simulink model "BMSModel"  (R2024b) (continued)
== system BMSModel/Electric Vehicul Model/Contactors == (continued)
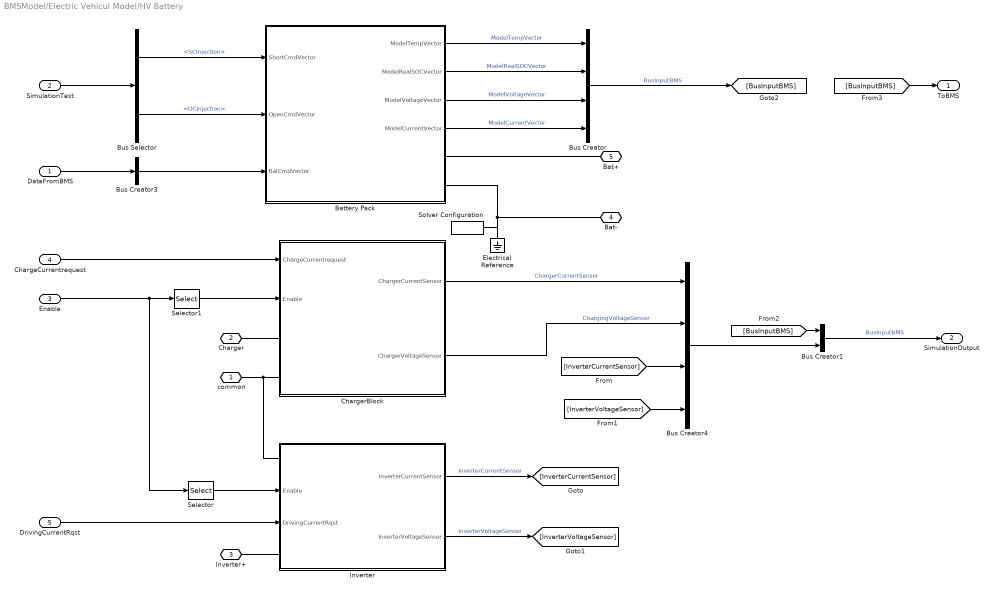
== system BMSModel/Electric Vehicul Model/HV Battery ==
  DataFromBMS : Inport
  SimulationTest : Inport  Port=2
  Enable : Inport  Port=3
  ChargeCurrentrequest : Inport  Port=4
  DrivingCurrentRqst : Inport  Port=5
  Battery Pack : Subsystem
  Bus Creator : BusCreator  Inputs=4
  Bus Creator1 : BusCreator  Inputs=2
  Bus Creator3 : BusCreator  Inputs=1
  Bus Creator4 : BusCreator  Inputs=4
  Bus Selector : BusSelector  OutputSignals=SimulationBatteryPackInput.SCInjection,SimulationBatteryPackInput.OCInjection
  ChargerBlock : Subsystem
  Electrical Reference : fl_lib/Electrical/Electrical Elements/Electrical Reference
  Inverter : Subsystem
      settings: PortSchema="{\"entries\":[{\"content\":{\"sides\":[{\"content\":{\"connectorIds\":[\"LConn1\",\"In1\",\"In2\",\"LConn2\"]},\"type\":\"ConnectorPlacement.EquallySpacedRectSide\",\"uuid\":\"3d4faee9-a6b8-4949-a26b-5ad71604b134\"},{\"content\":{\"connectorIds\":[\"Out1\",\"Out2\"],\"side\":\"RIGHT\"},\"type\":\"ConnectorPlacement.EquallySpacedRectSide\",\"uuid\":\"3f236c3c-2e31-4720-8762-4bdcf15f44c6\"},{\"content\":{\"side\":\"TOP\"},\"type\":\"ConnectorPlacement.EquallySpacedRectSide\",\"uuid\":\"f5349ad7-caad-4613-84e6-ed68add5efa8\"}]},\"type\":\"ConnectorPlacement.EquallySpacedConnector\",\"uuid\":\"6d42bc58-15ab-4454-81b3-fca0047b1a2e\"}],\"packageUris\":[\"http://schema.mathworks.com/mf0/Connector/R2020b\"],\"version\":\"1.0\"}"
  Selector : Selector  InputPortWidth=2  IndexOptions=Index vector (dialog)  Indices=2  OutputSizes=1
  Selector1 : Selector  InputPortWidth=2  IndexOptions=Index vector (dialog)  Indices=1  OutputSizes=1
  Solver Configuration : nesl_utility/Solver Configuration  PhysicalDomain=network_engine_domain  SubClassName=solver  LeftPortType=input  RightPortType=generic  Tones=[]  Harmonics=[]  SimulateNoise=off  Profile=off  UseLocalSampling=off  EnableSwitchedLinearOptims=on  FrequencyDomain=off  UseCCode=off  RelTol=0.001  AbsTol=1e-06  MinStep=1e-09  ToWorkspace=off  RevertToSquareIcSolve=off  NumHomotopyIterations=100  PhasorMode=off  PhasorModeNumHarmonics=1  ConsistencySolver=NEWTON_FTOL  IndexReductionMethod=DerivativeReplacement  DoDC=off  ResidualTolerance=1e-09  ConsistencyTolSource=GLOBAL  ConsistencyAbsTol=0.001  ConsistencyRelTol=0.001  ConsistencyTolFactor=0.001  UseLocalSolver=off  LocalSolverChoice=NE_BACKWARD_EULER_ADVANCER  ResolveIndetEquations=on  FunctionEvalNumThread=1  LocalSolverSampleTime=0.001  DoFixedCost=off  MaxNonlinIter=3  MaxModeIter=2  ComputeImpulses=off  ImpulseIterations=2  LinearAlgebra=auto  LinearAlgebraNumThread=0  EquationFormulation=NE_TIME_EF  DelaysMemoryBudget=1024  AutomaticFiltering=on  FilteringTimeConstant=0.001  PartitionStorageMethod=AS_NEEDED  PartitionMemoryBudget=1024  PartitionMethod=ROBUST  MultibodyUseLocalSolver=off  MultibodyLocalSolverChoice=ODE1  MultibodyLocalSolverSampleTime=0.001
  common : PMIOPort  Side=Left
  Charger : PMIOPort  Port=2  Side=Left
  Inverter+ : PMIOPort  Port=3  Side=Left
  Bat- : PMIOPort  Port=4  Side=Left
  Bat+ : PMIOPort  Port=5  Side=Left
  ToBMS : Outport
  SimulationOutput : Outport  Port=2
  wires:
  Battery Pack/2(ModelRealSOCVector) -> Bus Creator/2  "ModelRealSOCVector"
  Battery Pack/1(ModelTempVector) -> Bus Creator/1  "ModelTempVector"
  Bus Creator1/1 -> SimulationOutput/1  "BusInputBMS"
  Bus Selector/2 -> Battery Pack/2(OpenCmdVector)  "<OCInjection>"
  Battery Pack/4(ModelCurrentVector) -> Bus Creator/4  "ModelCurrentVector"
  Bus Selector/1 -> Battery Pack/1(ShortCmdVector)  "<SCInjection>"
  Battery Pack/3(ModelVoltageVector) -> Bus Creator/3  "ModelVoltageVector"
  Bus Creator/1 -> [BusInputBMS]  "BusInputBMS"
  SimulationTest/1 -> Bus Selector/1
  DataFromBMS/1 -> Bus Creator3/1
  Bus Creator3/1 -> Battery Pack/3(BalCmdVector)
  ChargerBlock/1(ChargerCurrentSensor) -> Bus Creator4/1  "ChargerCurrentSensor"
  ChargerBlock/2(ChargerVoltageSensor) -> Bus Creator4/2  "ChargingVoltageSensor"
  Inverter/1(InverterCurrentSensor) -> [InverterCurrentSensor]  "InverterCurrentSensor"
  Inverter/2(InverterVoltageSensor) -> [InverterVoltageSensor]  "InverterVoltageSensor"
  ChargeCurrentrequest/1 -> ChargerBlock/1(ChargeCurrentrequest)
  DrivingCurrentRqst/1 -> Inverter/2(DrivingCurrentRqst)
  Selector/1 -> Inverter/1(Enable)
  Selector1/1 -> ChargerBlock/2(Enable)
  Enable/1 -> Selector/1, Selector1/1
  Bus Creator4/1 -> Bus Creator1/2
  [InverterCurrentSensor] -> Bus Creator4/3
  [InverterVoltageSensor] -> Bus Creator4/4
  [BusInputBMS] -> Bus Creator1/1
  [BusInputBMS] -> ToBMS/1
  connections:
  Inverter/LConn1 -- common/RConn1 -- ChargerBlock/LConn2
  Charger/RConn1 -- ChargerBlock/LConn1
  Inverter+/RConn1 -- Inverter/LConn2
  Bat+/RConn1 -- Battery Pack/RConn1
  Bat-/RConn1 -- Solver Configuration/RConn1 -- Electrical Reference/LConn1 -- Battery Pack/RConn2

== system BMSModel/Test Senarois/Signal Builder ==
  Demux : Demux  Tag=STV Demux  Outputs=1
      settings: DisplayOption=none
  FromWs : FromWorkspace  Tag=STV FromWs  VariableName=tuvar
      SigBuilderData=|
      | common.afterFinalStr = 'Extrapolate'
      | common.sampleTime = 0
      | dataSet.timeRange = [0 2474]
      | dataSet.name = 'FTP-75 Drive Cycle'
      | sbobj.Groups.Name = 'FTP-75 Drive Cycle'
      | sbobj.Groups.Signals.Name = 'Current'
      | sbobj.Groups.Signals.XData = [0 0.00166966283167 0.00489865696245 0.00887290141926 0.01 0.0132228027433 0.0164456054866 0.0196684082299 0.02 0.0216579588505 0.0216579588505 0.023878217756 ... (264079 elements, 1x264079)]
      | sbobj.Groups.Signals.YData = [0 0 0 0 0 0 0 0 0 0 -7.47487403974e-31 -1.06202348499e-17 ... (264079 elements, 1x264079)]
      | sbobj.Groups.TimeRange = [0 2474]
  Current : Outport  Tag=STV Outport
  wires:
  FromWs/1 -> Demux/1
  Demux/1 -> Current/1

== system BMSModel/Electric Vehicul Model/BMS model/Balancing system ==
  SystemState : Inport
  VoltageVector : Inport  Port=2
  Balancing Function : MATLAB Function
      settings: MinAlgLoopOccurrences=on
  Data Type Conversion : DataTypeConversion  OutDataTypeStr=boolean  SaturateOnIntegerOverflow=off  RndMeth=Floor
  balancingCmd : Outport
  balancingDeltaVCell : Outport  Port=2
  balancingFlags : Outport  Port=3
  wires:
  SystemState/1 -> Balancing Function/1(systemState)
  VoltageVector/1 -> Balancing Function/2(voltageVector)
  Balancing Function/1(balancingCmd) -> Data Type Conversion/1
  Balancing Function/2(balancingFlags) -> balancingFlags/1
  Balancing Function/3(balancingDeltaVCell) -> balancingDeltaVCell/1
  Data Type Conversion/1 -> balancingCmd/1

== system BMSModel/Electric Vehicul Model/BMS model/Contactor commands ==
  SystemState : Inport
  ContactorModuleData : Inport  Port=2
  Bus Creator1 : BusCreator  Inputs=ContactorCmd,PreChrgCmd,DisChrgCmd,DrivetrainEn
  Bus Selector : BusSelector  OutputSignals=VCharger,VInverter,VBatt
  Chart : Stateflow chart  TreatAsAtomicUnit=on
  Data Type Conversion{,1..3} (4 alike) : DataTypeConversion  OutDataTypeStr=double  SaturateOnIntegerOverflow=off  RndMeth=Floor
  Vector Concatenate : Concatenate
  Vector Concatenate1 : Concatenate  NumInputs=3
  Vector Concatenate2 : Concatenate
  Vector Concatenate3 : Concatenate
  ContactorModuleCmd : Outport
  wires:
  Data Type Conversion2/1 -> Bus Creator1/3  "DisChrgCmd"
  Data Type Conversion/1 -> Bus Creator1/2  "PreChrgCmd"
  Vector Concatenate1/1 -> Data Type Conversion1/1
  Chart/4(preChargerCmd) -> Vector Concatenate/1
  Chart/7(disInverterCmd) -> Vector Concatenate2/2
  Vector Concatenate3/1 -> Data Type Conversion3/1
  Vector Concatenate/1 -> Data Type Conversion/1
  Chart/3(contCommonCmd) -> Vector Concatenate1/3
  Chart/6(disChargerCmd) -> Vector Concatenate2/1
  Vector Concatenate2/1 -> Data Type Conversion2/1
  Chart/9(driveEn) -> Vector Concatenate3/2
  SystemState/1 -> Chart/4(systemState)
  Chart/8(chargeEn) -> Vector Concatenate3/1
  Data Type Conversion3/1 -> Bus Creator1/4  "DrivetrainEn"
  Data Type Conversion1/1 -> Bus Creator1/1  "ContactorCmd"
  Chart/1(contChargerCmd) -> Vector Concatenate1/1
  Chart/5(preInverterCmd) -> Vector Concatenate/2
  Bus Creator1/1 -> ContactorModuleCmd/1
  Chart/2(contInverterCmd) -> Vector Concatenate1/2
  ContactorModuleData/1 -> Bus Selector/1
  Bus Selector/1 -> Chart/1(VCharger)  "<VCharger>"
  Bus Selector/2 -> Chart/2(VInverter)  "<VInverter>"
  Bus Selector/3 -> Chart/3(Vbatt)  "<VBatt>"

== system BMSModel/Electric Vehicul Model/BMS model/Faults detection ==
  <CurrentVector> : Inport
  <TempVector> : Inport  Port=2
  <VoltageVector> : Inport  Port=3
  Bus Creator2 : BusCreator  Inputs=8
  MATLAB Function : MATLAB Function  TreatAsAtomicUnit=on
  MATLAB Function1 : MATLAB Function  TreatAsAtomicUnit=on
  MATLAB Function2 : MATLAB Function  TreatAsAtomicUnit=on
  MATLAB Function3 : MATLAB Function  TreatAsAtomicUnit=on
  Max10 : MinMax  Function=max
  Max13 : MinMax
  Max4 : MinMax  Function=max
  Max7 : MinMax  Function=max
  BMSFaultOutput : Outport
  wires:
  Bus Creator2/1 -> BMSFaultOutput/1
  MATLAB Function2/1(W) -> Bus Creator2/3  "OverTemperatureWarning"
  Max4/1 -> MATLAB Function3/1(maxInput)
  <CurrentVector>/1 -> Max4/1
  MATLAB Function3/1(W) -> Bus Creator2/1  "OverCurrentWarning"
  MATLAB Function3/2(F) -> Bus Creator2/2  "OverCurrentFault"
  Max13/1 -> MATLAB Function/1(minInput)
  MATLAB Function1/2(F) -> Bus Creator2/6  "OverVoltageFault"
  MATLAB Function1/1(W) -> Bus Creator2/5  "OverVoltageWarning"
  <TempVector>/1 -> Max7/1
  Max10/1 -> MATLAB Function1/1(maxInput)
  <VoltageVector>/1 -> Max13/1, Max10/1
  Max7/1 -> MATLAB Function2/1(maxInput)
  MATLAB Function/1(W) -> Bus Creator2/7  "LowVoltageWarning"
  MATLAB Function/2(F) -> Bus Creator2/8  "LowVoltageFault"
  MATLAB Function2/2(F) -> Bus Creator2/4  "OverTemperatureFault"

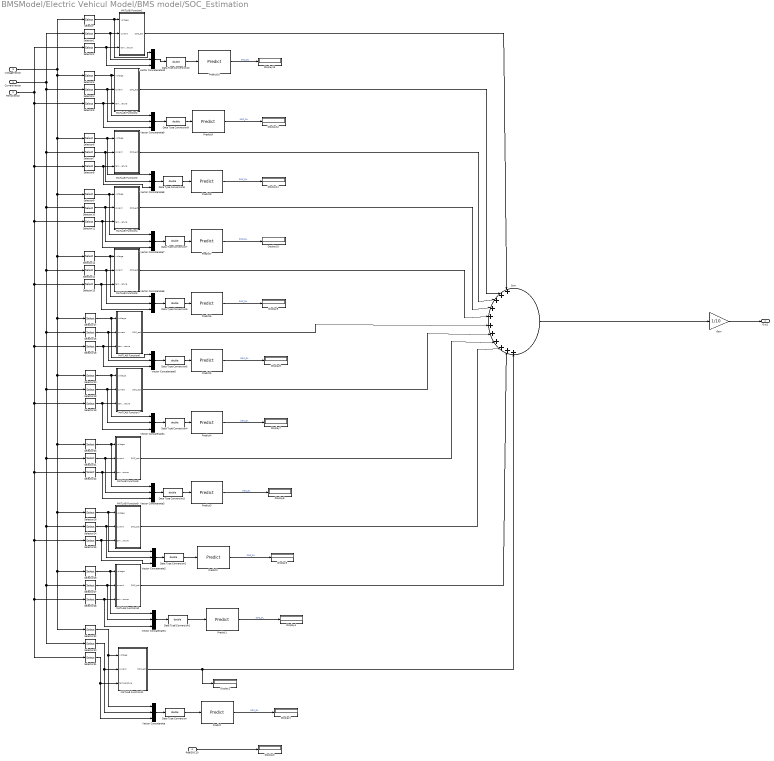
== system BMSModel/Electric Vehicul Model/BMS model/SOC_Estimation ==
  CurrentVector : Inport
  TempVector : Inport  Port=2
  VoltageVector : Inport  Port=3
  RealSOC10 : Inport  Port=4
  Data Type Conversion{,1..10} (11 alike) : DataTypeConversion  OutDataTypeStr=double  SaturateOnIntegerOverflow=off  RndMeth=Floor
  Display{1..13} (13 alike) : Display
  Gain : Gain  Gain=1/10
  MATLAB Function1 : MATLAB Function  TreatAsAtomicUnit=on
  MATLAB Function10 : MATLAB Function  TreatAsAtomicUnit=on
  MATLAB Function11 : MATLAB Function  TreatAsAtomicUnit=on
  MATLAB Function2 : MATLAB Function  TreatAsAtomicUnit=on
  MATLAB Function3 : MATLAB Function  TreatAsAtomicUnit=on
  MATLAB Function4 : MATLAB Function  TreatAsAtomicUnit=on
  MATLAB Function5 : MATLAB Function  TreatAsAtomicUnit=on
  MATLAB Function6 : MATLAB Function  TreatAsAtomicUnit=on
  MATLAB Function7 : MATLAB Function  TreatAsAtomicUnit=on
  MATLAB Function8 : MATLAB Function  TreatAsAtomicUnit=on
  MATLAB Function9 : MATLAB Function  TreatAsAtomicUnit=on
  Predict{,1..10} (11 alike) : deeplib/Predict  Network=Network from MAT-file  NetworkFunction=squeezenet  NetworkFilePath=D:\BMS-AI\Dev_BMS_with_AI\Outputs\nasa_lstmSOCNet_COMPLETE.mat  MiniBatchSize=128  InputDataFormats={'',''}  Predictions=on  Activations={}  ForceInterpretedSim=off  EnableDLNetwork=on  LastKnownNetworkFilePath=''  LastKnownNetworkTimeStamp=''
  Selector : Selector  InputPortWidth=10  IndexOptions=Index vector (dialog)  Indices=1  OutputSizes=1  RuntimeRangeChecks=on
  Selector1 : Selector  InputPortWidth=10  IndexOptions=Index vector (dialog)  Indices=1  OutputSizes=1  RuntimeRangeChecks=on
  Selector{6..11} (6 alike) : Selector  InputPortWidth=10  IndexOptions=Index vector (dialog)  Indices=3  OutputSizes=1  RuntimeRangeChecks=on
  Selector12 : Selector  InputPortWidth=10  IndexOptions=Index vector (dialog)  Indices=4  OutputSizes=1  RuntimeRangeChecks=on
  Selector13 : Selector  InputPortWidth=10  IndexOptions=Index vector (dialog)  Indices=4  OutputSizes=1  RuntimeRangeChecks=on
  Selector14 : Selector  InputPortWidth=10  IndexOptions=Index vector (dialog)  Indices=4  OutputSizes=1  RuntimeRangeChecks=on
  Selector15 : Selector  InputPortWidth=10  IndexOptions=Index vector (dialog)  Indices=5  OutputSizes=1  RuntimeRangeChecks=on
  Selector16 : Selector  InputPortWidth=10  IndexOptions=Index vector (dialog)  Indices=5  OutputSizes=1  RuntimeRangeChecks=on
  Selector17 : Selector  InputPortWidth=10  IndexOptions=Index vector (dialog)  Indices=5  OutputSizes=1  RuntimeRangeChecks=on
  Selector18 : Selector  InputPortWidth=10  IndexOptions=Index vector (dialog)  Indices=6  OutputSizes=1  RuntimeRangeChecks=on
  Selector19 : Selector  InputPortWidth=10  IndexOptions=Index vector (dialog)  Indices=6  OutputSizes=1  RuntimeRangeChecks=on
  Selector2 : Selector  InputPortWidth=10  IndexOptions=Index vector (dialog)  Indices=1  OutputSizes=1  RuntimeRangeChecks=on
  Selector20 : Selector  InputPortWidth=10  IndexOptions=Index vector (dialog)  Indices=6  OutputSizes=1  RuntimeRangeChecks=on
  Selector21 : Selector  InputPortWidth=10  IndexOptions=Index vector (dialog)  Indices=7  OutputSizes=1  RuntimeRangeChecks=on
  Selector22 : Selector  InputPortWidth=10  IndexOptions=Index vector (dialog)  Indices=7  OutputSizes=1  RuntimeRangeChecks=on
  Selector23 : Selector  InputPortWidth=10  IndexOptions=Index vector (dialog)  Indices=7  OutputSizes=1  RuntimeRangeChecks=on
  Selector24 : Selector  InputPortWidth=10  IndexOptions=Index vector (dialog)  Indices=8  OutputSizes=1  RuntimeRangeChecks=on
  Selector25 : Selector  InputPortWidth=10  IndexOptions=Index vector (dialog)  Indices=8  OutputSizes=1  RuntimeRangeChecks=on
  Selector26 : Selector  InputPortWidth=10  IndexOptions=Index vector (dialog)  Indices=8  OutputSizes=1  RuntimeRangeChecks=on
  Selector27 : Selector  InputPortWidth=10  IndexOptions=Index vector (dialog)  Indices=9  OutputSizes=1  RuntimeRangeChecks=on
  Selector28 : Selector  InputPortWidth=10  IndexOptions=Index vector (dialog)  Indices=9  OutputSizes=1  RuntimeRangeChecks=on
  Selector29 : Selector  InputPortWidth=10  IndexOptions=Index vector (dialog)  Indices=9  OutputSizes=1  RuntimeRangeChecks=on
  Selector3 : Selector  InputPortWidth=10  IndexOptions=Index vector (dialog)  Indices=2  OutputSizes=1  RuntimeRangeChecks=on
  Selector30 : Selector  InputPortWidth=10  IndexOptions=Index vector (dialog)  Indices=10  OutputSizes=1  RuntimeRangeChecks=on
  Selector31 : Selector  InputPortWidth=10  IndexOptions=Index vector (dialog)  Indices=10  OutputSizes=1  RuntimeRangeChecks=on
  Selector32 : Selector  InputPortWidth=10  IndexOptions=Index vector (dialog)  Indices=10  OutputSizes=1  RuntimeRangeChecks=on
  Selector4 : Selector  InputPortWidth=10  IndexOptions=Index vector (dialog)  Indices=2  OutputSizes=1  RuntimeRangeChecks=on
  Selector5 : Selector  InputPortWidth=10  IndexOptions=Index vector (dialog)  Indices=2  OutputSizes=1  RuntimeRangeChecks=on
  Sum : Sum  Inputs=|+++++++++++
  Vector Concatenate{,1..10} (11 alike) : Concatenate  NumInputs=3
  Out1 : Outport
  wires:
  Predict/1 -> Display1/1  "SOC_DL"
  Selector6/1 -> Vector Concatenate8/1, MATLAB Function3/1(voltage)
  Selector32/1 -> MATLAB Function11/1(voltage), Vector Concatenate/1
  Selector30/1 -> Vector Concatenate/2, MATLAB Function11/2(current)
  Selector31/1 -> Vector Concatenate/3, MATLAB Function11/3(temperature)
  TempVector/1 -> Selector5/1, Selector8/1, Selector11/1, Selector13/1, Selector16/1, Selector19/1, Selector22/1, Selector25/1, Selector28/1, Selector31/1, Selector2/1
  CurrentVector/1 -> Selector1/1, Selector4/1, Selector7/1, Selector10/1, Selector12/1, Selector15/1, Selector18/1, Selector21/1, Selector24/1, Selector27/1, Selector30/1
  VoltageVector/1 -> Selector/1, Selector3/1, Selector6/1, Selector9/1, Selector14/1, Selector17/1, Selector20/1, Selector23/1, Selector29/1, Selector32/1, Selector26/1
  MATLAB Function1/1(SOC_est) -> Sum/1
  MATLAB Function2/1(SOC_est) -> Sum/2
  MATLAB Function3/1(SOC_est) -> Sum/3
  MATLAB Function4/1(SOC_est) -> Sum/4
  MATLAB Function5/1(SOC_est) -> Sum/5
  MATLAB Function6/1(SOC_est) -> Sum/6
  MATLAB Function7/1(SOC_est) -> Sum/7
  MATLAB Function8/1(SOC_est) -> Sum/8
  MATLAB Function9/1(SOC_est) -> Sum/9
  MATLAB Function10/1(SOC_est) -> Sum/10
  MATLAB Function11/1(SOC_est) -> Display2/1, Sum/11
  Sum/1 -> Gain/1
  Gain/1 -> Out1/1
  Vector Concatenate/1 -> Data Type Conversion/1
  Data Type Conversion/1 -> Predict/1
  RealSOC10/1 -> Display3/1
  Selector28/1 -> MATLAB Function10/3(temperature), Vector Concatenate1/3
  Vector Concatenate1/1 -> Data Type Conversion1/1
  Data Type Conversion1/1 -> Predict1/1
  Selector29/1 -> MATLAB Function10/1(voltage), Vector Concatenate1/1
  Selector27/1 -> MATLAB Function10/2(current), Vector Concatenate1/2
  Predict1/1 -> Display4/1  "SOC_DL"
  Selector25/1 -> Vector Concatenate2/3, MATLAB Function9/3(temperature)
  Vector Concatenate2/1 -> Data Type Conversion2/1
  Predict2/1 -> Display5/1  "SOC_DL"
  Selector24/1 -> Vector Concatenate2/2, MATLAB Function9/2(current)
  Selector26/1 -> Vector Concatenate2/1, MATLAB Function9/1(voltage)
  Data Type Conversion2/1 -> Predict2/1
  Selector22/1 -> Vector Concatenate3/3, MATLAB Function8/3(temperature)
  Vector Concatenate3/1 -> Data Type Conversion3/1
  Predict3/1 -> Display6/1  "SOC_DL"
  Selector21/1 -> Vector Concatenate3/2, MATLAB Function8/2(current)
  Selector23/1 -> Vector Concatenate3/1, MATLAB Function8/1(voltage)
  Data Type Conversion3/1 -> Predict3/1
  Selector19/1 -> Vector Concatenate4/3, MATLAB Function7/3(temperature)
  Vector Concatenate4/1 -> Data Type Conversion4/1
  Predict4/1 -> Display7/1  "SOC_DL"
  Selector18/1 -> Vector Concatenate4/2, MATLAB Function7/2(current)
  Selector20/1 -> Vector Concatenate4/1, MATLAB Function7/1(voltage)
  Data Type Conversion4/1 -> Predict4/1
  Selector16/1 -> Vector Concatenate5/3, MATLAB Function6/3(temperature)
  Vector Concatenate5/1 -> Data Type Conversion5/1
  Predict5/1 -> Display8/1  "SOC_DL"
  Selector15/1 -> Vector Concatenate5/2, MATLAB Function6/2(current)
  Selector17/1 -> Vector Concatenate5/1, MATLAB Function6/1(voltage)
  Data Type Conversion5/1 -> Predict5/1
  Selector13/1 -> Vector Concatenate6/3, MATLAB Function5/3(temperature)
  Vector Concatenate6/1 -> Data Type Conversion6/1
  Predict6/1 -> Display9/1  "SOC_DL"
  Selector12/1 -> Vector Concatenate6/2, MATLAB Function5/2(current)
  Selector14/1 -> Vector Concatenate6/1, MATLAB Function5/1(voltage)
  Data Type Conversion6/1 -> Predict6/1
  Selector11/1 -> Vector Concatenate7/3, MATLAB Function4/3(temperature)
  Vector Concatenate7/1 -> Data Type Conversion7/1
  Predict7/1 -> Display10/1  "SOC_DL"
  Selector10/1 -> Vector Concatenate7/2, MATLAB Function4/2(current)
  Selector9/1 -> Vector Concatenate7/1, MATLAB Function4/1(voltage)
  Data Type Conversion7/1 -> Predict7/1
  Selector8/1 -> Vector Concatenate8/3, MATLAB Function3/3(temperature)
  Vector Concatenate8/1 -> Data Type Conversion8/1
  Predict8/1 -> Display11/1  "SOC_DL"
  Selector7/1 -> Vector Concatenate8/2, MATLAB Function3/2(current)
  Data Type Conversion8/1 -> Predict8/1
  Selector5/1 -> Vector Concatenate9/3, MATLAB Function2/3(temperature)
  Vector Concatenate9/1 -> Data Type Conversion9/1
  Predict9/1 -> Display12/1  "SOC_DL"
  Selector4/1 -> Vector Concatenate9/2, MATLAB Function2/2(current)
  Selector3/1 -> Vector Concatenate9/1, MATLAB Function2/1(voltage)
  Data Type Conversion9/1 -> Predict9/1
  Selector2/1 -> Vector Concatenate10/3, MATLAB Function1/3(temperature)
  Vector Concatenate10/1 -> Data Type Conversion10/1
  Predict10/1 -> Display13/1  "SOC_DL"
  Selector1/1 -> Vector Concatenate10/2, MATLAB Function1/2(current)
  Selector/1 -> Vector Concatenate10/1, MATLAB Function1/1(voltage)
  Data Type Conversion10/1 -> Predict10/1

== system BMSModel/Electric Vehicul Model/HV Battery/Battery Pack ==
  ShortCmdVector : Inport
  OpenCmdVector : Inport  Port=2
  BalCmdVector : Inport  Port=3
  Battery Model : Subsystem
      settings: PortSchema="{\"entries\":[{\"content\":{\"sides\":[{\"content\":{\"connectorIds\":[\"In1\",\"In2\",\"In3\",\"In4\"]},\"type\":\"ConnectorPlacement.EquallySpacedRectSide\",\"uuid\":\"339fc2dc-73de-410a-a97e-1fe159b417b4\"},{\"content\":{\"connectorIds\":[\"RConn1\",\"Out1\",\"Out2\",\"Out3\",\"Out4\",\"RConn2\"],\"side\":\"RIGHT\"},\"type\":\"ConnectorPlacement.EquallySpacedRectSide\",\"uuid\":\"1ff17677-7e7c-48aa-9b47-364d19db4b22\"},{\"content\":{\"side\":\"TOP\"},\"type\":\"ConnectorPlacement.EquallySpacedRectSide\",\"uuid\":\"a6a1d56b-2099-4034-99b1-512debfe3694\"}]},\"type\":\"ConnectorPlacement.EquallySpacedConnector\",\"uuid\":\"fb04511b-02af-41ab-983c-5fc3ec8a5a5f\"}],\"packageUris\":[\"http://schema.mathworks.com/mf0/Connector/R2020b\"],\"version\":\"1.0\"}"
  Constant : Constant  Value=25
  Bat+ : PMIOPort  Side=Right
  BattMod- : PMIOPort  Port=2  Side=Right
  ModelTempVector : Outport
  ModelRealSOCVector : Outport  Port=2
  ModelVoltageVector : Outport  Port=3
  ModelCurrentVector : Outport  Port=4
  wires:
  Battery Model/1(TCellVector) -> [TCellVector1]  "Temp"
  [TCellVector1] -> ModelTempVector/1
  Constant/1 -> Battery Model/1(AmbTemp)
  ShortCmdVector/1 -> Battery Model/2(ShortFaultCmd)
  [CurrentVector1] -> ModelCurrentVector/1
  [RealSOCVector1] -> ModelRealSOCVector/1
  OpenCmdVector/1 -> Battery Model/3(OpenFaultCmd)
  [VoltageVector1] -> ModelVoltageVector/1
  Battery Model/2(RealSOCVector) -> [RealSOCVector1]
  BalCmdVector/1 -> Battery Model/4(PassiveControlBalCmd)
  Battery Model/3(VoltageVector) -> [VoltageVector1]
  Battery Model/4(CurrentCellVector) -> [CurrentVector1]
  connections:
  Battery Model/RConn1 -- Bat+/RConn1
  BattMod-/RConn1 -- Battery Model/RConn2

== system BMSModel/Electric Vehicul Model/HV Battery/ChargerBlock ==
  ChargeCurrentrequest : Inport
  Enable : Inport  Port=2
  Cast To Double : DataTypeConversion  OutDataTypeStr=double  SaturateOnIntegerOverflow=off  RndMeth=Floor
  Cast To Double1 : DataTypeConversion  OutDataTypeStr=double  SaturateOnIntegerOverflow=off  RndMeth=Floor
  Cast To Double2 : DataTypeConversion  OutDataTypeStr=double  SaturateOnIntegerOverflow=off  RndMeth=Floor
  ChargerCap : fl_lib/Electrical/Electrical Elements/Capacitor  c=ChargerCap F  r=1e-6 Ohm  g=0 1/Ohm  i_specify=off  i_priority=None  i=0 A  i_nominal_specify=off  i_nominal_value=1  i_nominal_unit=A  v_specify=off  v_priority=None  v=0 V  v_nominal_specify=off  v_nominal_value=1  v_nominal_unit=V  vc_specify=off  vc_priority=High  vc=0 V  vc_nominal_specify=off  vc_nominal_value=1  vc_nominal_unit=V
  ChargerParResistance : fl_lib/Electrical/Electrical Elements/Resistor  R=ChargerParRes Ohm  i_specify=off  i_priority=None  i=0 A  i_nominal_specify=off  i_nominal_value=1  i_nominal_unit=A  v_specify=off  v_priority=None  v=0 V  v_nominal_specify=off  v_nominal_value=1  v_nominal_unit=V
  ChargerResistance : fl_lib/Electrical/Electrical Elements/Resistor  R=ChargerRes Ohm  i_specify=off  i_priority=None  i=0 A  i_nominal_specify=off  i_nominal_value=1  i_nominal_unit=A  v_specify=off  v_priority=None  v=0 V  v_nominal_specify=off  v_nominal_value=1  v_nominal_unit=V
  Constant3 : Constant  Value=0
  Controlled Current Source1 : fl_lib/Electrical/Electrical Sources/Controlled Current Source
  Current Sensor1 : fl_lib/Electrical/Electrical Sensors/Current Sensor
  PS-Simulink Converter : nesl_utility/PS-Simulink Converter  PhysicalDomain=network_engine_domain  SubClassName=ps_output  LeftPortType=input  RightPortType=output  PseudoPeriodic=off  Frequencies=[]  VectorFormat=1-D array  Unit=inherit  AffineConversion=off
  PS-Simulink Converter1 : nesl_utility/PS-Simulink Converter  PhysicalDomain=network_engine_domain  SubClassName=ps_output  LeftPortType=input  RightPortType=output  PseudoPeriodic=off  Frequencies=[]  VectorFormat=1-D array  Unit=inherit  AffineConversion=off
  Simulink-PS Converter1 : nesl_utility/Simulink-PS Converter  PhysicalDomain=network_engine_domain  SubClassName=ps_input  LeftPortType=input  RightPortType=output  PseudoPeriodic=off  Frequencies=[]  NoiseDistribution=none  NoiseParameters=[]  Unit=1  AffineConversion=off  FilteringAndDerivatives=provide  SimscapeFilterOrder=1  InputFilterTimeConstant=0.001  UdotUserProvided=0
  Switch1 : Switch  Criteria=u2 > Threshold  SaturateOnIntegerOverflow=off  InputSameDT=off
  Voltage Sensor1 : fl_lib/Electrical/Electrical Sensors/Voltage Sensor
  Charger+ : PMIOPort  Side=Left
  Charger- : PMIOPort  Port=2  Side=Left
  ChargerCurrentSensor : Outport
  ChargerVoltageSensor : Outport  Port=2
  wires:
  Cast To Double/1 -> Simulink-PS Converter1/1
  Constant3/1 -> Switch1/3
  PS-Simulink Converter/1 -> Cast To Double1/1
  PS-Simulink Converter1/1 -> Cast To Double2/1
  Cast To Double1/1 -> ChargerCurrentSensor/1
  Cast To Double2/1 -> ChargerVoltageSensor/1
  Enable/1 -> Switch1/2
  ChargeCurrentrequest/1 -> Switch1/1
  Switch1/1 -> Cast To Double/1
  connections:
  Controlled Current Source1/LConn1 -- Current Sensor1/LConn1
  Voltage Sensor1/LConn1 -- ChargerResistance/RConn1 -- Current Sensor1/RConn2
  Simulink-PS Converter1/RConn1 -- Controlled Current Source1/RConn1
  ChargerCap/LConn1 -- Charger+/RConn1 -- ChargerParResistance/LConn1 -- ChargerResistance/LConn1
  PS-Simulink Converter1/LConn1 -- Voltage Sensor1/RConn1
  Controlled Current Source1/RConn2 -- Charger-/RConn1 -- ChargerCap/RConn1 -- ChargerParResistance/RConn1 -- Voltage Sensor1/RConn2
  Current Sensor1/RConn1 -- PS-Simulink Converter/LConn1

== system BMSModel/Electric Vehicul Model/HV Battery/Inverter ==
  Enable : Inport
  DrivingCurrentRqst : Inport  Port=2
  Cast To Double : DataTypeConversion  OutDataTypeStr=double  SaturateOnIntegerOverflow=off  RndMeth=Floor
  Cast To Double1 : DataTypeConversion  OutDataTypeStr=double  SaturateOnIntegerOverflow=off  RndMeth=Floor
  Cast To Double2 : DataTypeConversion  OutDataTypeStr=double  SaturateOnIntegerOverflow=off  RndMeth=Floor
  Constant3 : Constant  Value=0
  Controlled Current Source1 : fl_lib/Electrical/Electrical Sources/Controlled Current Source
  Current Sensor1 : fl_lib/Electrical/Electrical Sensors/Current Sensor
  InverterCap : fl_lib/Electrical/Electrical Elements/Capacitor  c=InverterCap F  r=1e-6 Ohm  g=0 1/Ohm  i_specify=off  i_priority=None  i=0 A  i_nominal_specify=off  i_nominal_value=1  i_nominal_unit=A  v_specify=off  v_priority=None  v=0 V  v_nominal_specify=off  v_nominal_value=1  v_nominal_unit=V  vc_specify=off  vc_priority=High  vc=0 V  vc_nominal_specify=off  vc_nominal_value=1  vc_nominal_unit=V
  InverterParResistance : fl_lib/Electrical/Electrical Elements/Resistor  R=InverterParRes Ohm  i_specify=off  i_priority=None  i=0 A  i_nominal_specify=off  i_nominal_value=1  i_nominal_unit=A  v_specify=off  v_priority=None  v=0 V  v_nominal_specify=off  v_nominal_value=1  v_nominal_unit=V
  InverterResistance : fl_lib/Electrical/Electrical Elements/Resistor  R=InverterRes Ohm  i_specify=off  i_priority=None  i=0 A  i_nominal_specify=off  i_nominal_value=1  i_nominal_unit=A  v_specify=off  v_priority=None  v=0 V  v_nominal_specify=off  v_nominal_value=1  v_nominal_unit=V
  PS-Simulink Converter : nesl_utility/PS-Simulink Converter  PhysicalDomain=network_engine_domain  SubClassName=ps_output  LeftPortType=input  RightPortType=output  PseudoPeriodic=off  Frequencies=[]  VectorFormat=1-D array  Unit=inherit  AffineConversion=off
  PS-Simulink Converter1 : nesl_utility/PS-Simulink Converter  PhysicalDomain=network_engine_domain  SubClassName=ps_output  LeftPortType=input  RightPortType=output  PseudoPeriodic=off  Frequencies=[]  VectorFormat=1-D array  Unit=inherit  AffineConversion=off
  Simulink-PS Converter1 : nesl_utility/Simulink-PS Converter  PhysicalDomain=network_engine_domain  SubClassName=ps_input  LeftPortType=input  RightPortType=output  PseudoPeriodic=off  Frequencies=[]  NoiseDistribution=none  NoiseParameters=[]  Unit=1  AffineConversion=off  FilteringAndDerivatives=provide  SimscapeFilterOrder=1  InputFilterTimeConstant=0.001  UdotUserProvided=0
  Switch1 : Switch  Criteria=u2 > Threshold  SaturateOnIntegerOverflow=off  InputSameDT=off
  Voltage Sensor1 : fl_lib/Electrical/Electrical Sensors/Voltage Sensor
  Inverter- : PMIOPort  Side=Left
  Inverter+ : PMIOPort  Port=2  Side=Left
  InverterCurrentSensor : Outport
  InverterVoltageSensor : Outport  Port=2
  wires:
  Cast To Double/1 -> Simulink-PS Converter1/1
  Constant3/1 -> Switch1/3
  PS-Simulink Converter/1 -> Cast To Double1/1
  PS-Simulink Converter1/1 -> Cast To Double2/1
  Switch1/1 -> Cast To Double/1
  DrivingCurrentRqst/1 -> Switch1/1
  Cast To Double1/1 -> InverterCurrentSensor/1
  Cast To Double2/1 -> InverterVoltageSensor/1
  Enable/1 -> Switch1/2
  connections:
  Controlled Current Source1/RConn2 -- Current Sensor1/LConn1
  Voltage Sensor1/RConn2 -- InverterParResistance/RConn1 -- Inverter-/RConn1 -- InverterCap/RConn1 -- Controlled Current Source1/LConn1
  Simulink-PS Converter1/RConn1 -- Controlled Current Source1/RConn1
  InverterCap/LConn1 -- Inverter+/RConn1 -- InverterParResistance/LConn1 -- InverterResistance/LConn1
  InverterResistance/RConn1 -- Voltage Sensor1/LConn1 -- Current Sensor1/RConn2
  PS-Simulink Converter/LConn1 -- Current Sensor1/RConn1
  PS-Simulink Converter1/LConn1 -- Voltage Sensor1/RConn1
